simulink model "ClimaStopStrtReqMgr"  (R2022b)
settings: Solver=FixedStepDiscrete  StartTime=0.0  StopTime=10.0  FixedStep=1  RelTol=1e-3
settings Model: Creator=blue.ge  LastModifiedBy=blue.ge
settings DataIOCC: SaveFormat=StructureWithTime
settings OptimizationCC: DefaultParameterBehavior=Inlined  EnhancedBackFolding=on  PassReuseOutputArgsAs=Individual arguments  ZeroExternalMemoryAtStartup=off  ZeroInternalMemoryAtStartup=off  OptimizeBlockOrder=speed  DifferentSizesBufferReuse=on  OptimizationCustomize=off  EfficientTunableParamExpr=on
settings DebuggingCC: ParameterTunabilityLossMsg=error
settings ModelReferenceCC: ModelReferenceNumInstancesAllowed=Single
settings SFSimCC: MATLABDynamicMemAlloc=off
settings RTWCC: Description=AUTOSAR  SystemTargetFile=autosar.tlc  GenCodeOnly=on  TemplateMakefile=ert_default_tmf
settings CodeAppCC: ForceParamTrailComments=on  IgnoreCustomStorageClasses=off  OperatorAnnotations=on  SimulinkDataObjDesc=on  SFDataObjDesc=on  InsertBlockDesc=on
settings STFCustomTargetCC: TargetLangStandard=C99 (ISO)  MultiwordLength=256  ParMdlRefBuildCompliant=on  CompOptLevelCompliant=on  IncludeMdlTerminateFcn=off  CombineOutputUpdateFcns=on  SuppressErrorStatus=on  ERTCustomFileBanners=on  SupportNonFinite=off  SupportComplex=off  SupportContinuousTime=off  SupportNonInlinedSFcns=off  RemoveResetFunc=on  MATLABClassNameForMDSCustomization=Simulink.SoftwareTarget.ERTCustomization  AutosarCompliant=on  UseToolchainInfoCompliant=on  LookupTableObjectStructAxisOrder=2,1,3,4,...  LUTObjectStructOrderEvenSpacing=Size,Table,Breakpoints  SystemTargetFile=autosar.tlc
settings ERTTargetCC: TargetLangStandard=C99 (ISO)  ConcurrentExecutionCompliant=off  IncludeMdlTerminateFcn=off  SuppressErrorStatus=on  SupportNonFinite=off  SupportComplex=off  ModelStepFunctionPrototypeControlCompliant=off  CPPClassGenCompliant=off  AutosarCompliant=on  LookupTableObjectStructAxisOrder=2,1,3,4,...  LUTObjectStructOrderEvenSpacing=Size,Table,Breakpoints  InlinedParameterPlacement=NonHierarchical  ERTCustomFileTemplate=example_file_process.tlc

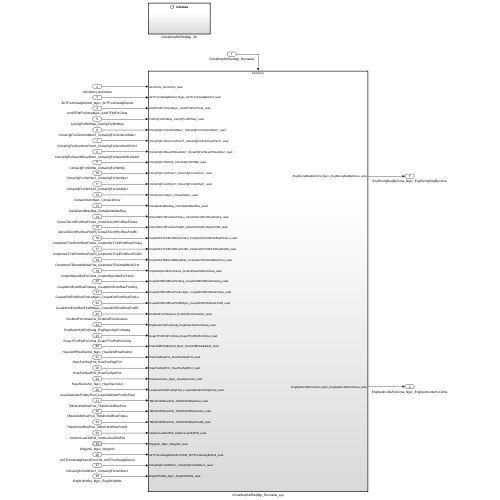
[thumbnail: Simulink's stored preview of the top-level diagram]
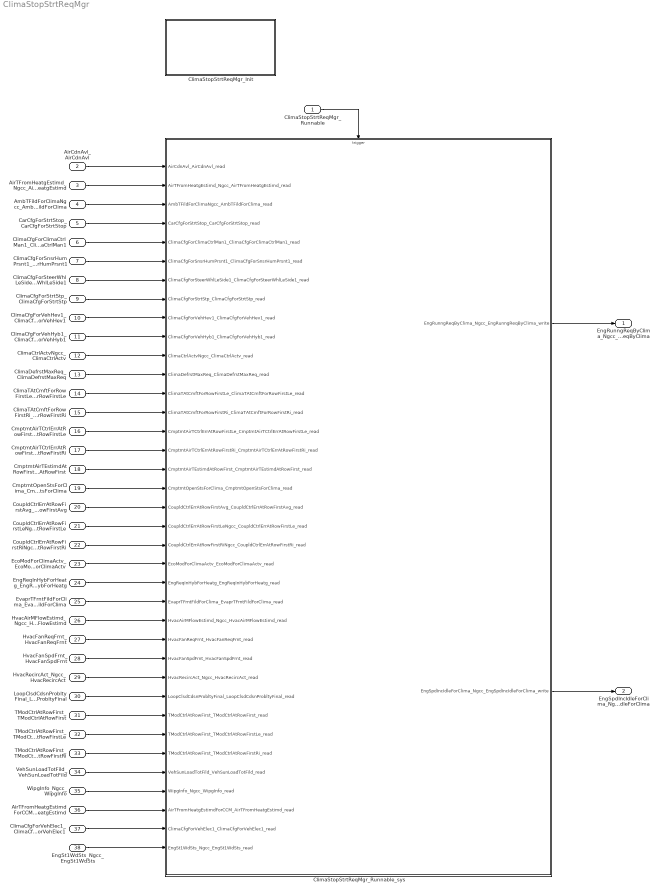
== system ClimaStopStrtReqMgr ==
  ClimaStopStrtReqMgr_Runnable : Inport  SampleTime=1  OutputFunctionCall=on
  AirCdnAvl_AirCdnAvl : Inport  Port=2  OutDataTypeStr=Boolean  PortDimensions=1
  AirTFromHeatgEstimd_Ngcc_AirTFromHeatgEstimd : Inport  Port=3  OutDataTypeStr=T30  PortDimensions=1
  AmbTFildForClimaNgcc_AmbTFildForClima : Inport  Port=4  OutDataTypeStr=T30  PortDimensions=1
  CarCfgForStrtStop_CarCfgForStrtStop : Inport  Port=5  OutDataTypeStr=Enum: CarCfgForStrtStop  PortDimensions=1
  ClimaCfgForClimaCtrlMan1_ClimaCfgForClimaCtrlMan1 : Inport  Port=6  OutDataTypeStr=Boolean  PortDimensions=1
  ClimaCfgForSnsrHumPrsnt1_ClimaCfgForSnsrHumPrsnt1 : Inport  Port=7  OutDataTypeStr=Boolean  PortDimensions=1
  ClimaCfgForSteerWhlLeSide1_ClimaCfgForSteerWhlLeSide1 : Inport  Port=8  OutDataTypeStr=Boolean  PortDimensions=1
  ClimaCfgForStrtStp_ClimaCfgForStrtStp : Inport  Port=9  OutDataTypeStr=Enum: CarCfgForStrtStop  PortDimensions=1
  ClimaCfgForVehHev1_ClimaCfgForVehHev1 : Inport  Port=10  OutDataTypeStr=Boolean  PortDimensions=1
  ClimaCfgForVehHyb1_ClimaCfgForVehHyb1 : Inport  Port=11  OutDataTypeStr=Boolean  PortDimensions=1
  ClimaCtrlActvNgcc_ClimaCtrlActv : Inport  Port=12  OutDataTypeStr=Boolean  PortDimensions=1
  ClimaDefrstMaxReq_ClimaDefrstMaxReq : Inport  Port=13  OutDataTypeStr=Boolean  PortDimensions=1
  ClimaTAtCmftForRowFirstLe_ClimaTAtCmftForRowFirstLe : Inport  Port=14  OutDataTypeStr=T30  PortDimensions=1
  ClimaTAtCmftForRowFirstRi_ClimaTAtCmftForRowFirstRi : Inport  Port=15  OutDataTypeStr=T30  PortDimensions=1
  CmptmtAirTCtrlErrAtRowFirstLe_CmptmtAirTCtrlErrAtRowFirstLe : Inport  Port=16  OutDataTypeStr=T30  PortDimensions=1
  CmptmtAirTCtrlErrAtRowFirstRi_CmptmtAirTCtrlErrAtRowFirstRi : Inport  Port=17  OutDataTypeStr=T30  PortDimensions=1
  CmptmtAirTEstimdAtRowFirst_CmptmtAirTEstimdAtRowFirst : Inport  Port=18  OutDataTypeStr=T30  PortDimensions=1
  CmptmtOpenStsForClima_CmptmtOpenStsForClima : Inport  Port=19  OutDataTypeStr=Boolean  PortDimensions=1
  CoupldCtrlErrAtRowFirstAvg_CoupldCtrlErrAtRowFirstAvg : Inport  Port=20  OutDataTypeStr=T30  PortDimensions=1
  CoupldCtrlErrAtRowFirstLeNgcc_CoupldCtrlErrAtRowFirstLe : Inport  Port=21  OutDataTypeStr=T30  PortDimensions=1
  CoupldCtrlErrAtRowFirstRiNgcc_CoupldCtrlErrAtRowFirstRi : Inport  Port=22  OutDataTypeStr=T30  PortDimensions=1
  EcoModForClimaActv_EcoModForClimaActv : Inport  Port=23  OutDataTypeStr=Enum: OnOff1  PortDimensions=1
  EngReqInHybForHeatg_EngReqInHybForHeatg : Inport  Port=24  OutDataTypeStr=Boolean  PortDimensions=1
  EvaprTFrntFildForClima_EvaprTFrntFildForClima : Inport  Port=25  OutDataTypeStr=T30  PortDimensions=1
  HvacAirMFlowEstimd_Ngcc_HvacAirMFlowEstimd : Inport  Port=26  OutMin=0  OutMax=1000  OutDataTypeStr=MFlow1  PortDimensions=1
  HvacFanReqFrnt_HvacFanReqFrnt : Inport  Port=27  OutDataTypeStr=HvacFanSpd  PortDimensions=1
  HvacFanSpdFrnt_HvacFanSpdFrnt : Inport  Port=28  OutDataTypeStr=HvacFanSpd  PortDimensions=1
  HvacRecircAct_Ngcc_HvacRecircAct : Inport  Port=29  OutDataTypeStr=Perc4  PortDimensions=1
  LoopClsdCdsnProbltyFinal_LoopClsdCdsnProbltyFinal : Inport  Port=30  OutDataTypeStr=Fac30  PortDimensions=1
  TModCtrlAtRowFirst_TModCtrlAtRowFirst : Inport  Port=31  OutDataTypeStr=T31  PortDimensions=1
  TModCtrlAtRowFirst_TModCtrlAtRowFirstLe : Inport  Port=32  OutDataTypeStr=T31  PortDimensions=1
  TModCtrlAtRowFirst_TModCtrlAtRowFirstRi : Inport  Port=33  OutDataTypeStr=T31  PortDimensions=1
  VehSunLoadTotFild_VehSunLoadTotFild : Inport  Port=34  OutDataTypeStr=SunLoad30  PortDimensions=1
  WipgInfo_Ngcc_WipgInfo : Inport  Port=35  OutDataTypeStr=Bus: WipgInfo  PortDimensions=1  BusOutputAsStruct=on
  AirTFromHeatgEstimdForCCM_AirTFromHeatgEstimd : Inport  Port=36  OutDataTypeStr=T30  PortDimensions=1
  ClimaCfgForVehElec1_ClimaCfgForVehElec1 : Inport  Port=37  OutDataTypeStr=Boolean  PortDimensions=1
  EngSt1WdSts_Ngcc_EngSt1WdSts : Inport  Port=38  OutDataTypeStr=Enum: EngSt1  PortDimensions=1
  ClimaStopStrtReqMgr_Init : Subsystem
  ClimaStopStrtReqMgr_Runnable_sys : Subsystem
  EngRunngReqByClima_Ngcc_EngRunngReqByClima : Outport  OutDataTypeStr=Enum: EngRunngReq  PortDimensions=1
  EngSpdIncIdleForClima_Ngcc_EngSpdIncIdleForClima : Outport  Port=2  OutDataTypeStr=Enum: Flg1  PortDimensions=1
  wires:
  AirCdnAvl_AirCdnAvl/1 -> ClimaStopStrtReqMgr_Runnable_sys/1(AirCdnAvl_AirCdnAvl_read)
  AirTFromHeatgEstimd_Ngcc_AirTFromHeatgEstimd/1 -> ClimaStopStrtReqMgr_Runnable_sys/2(AirTFromHeatgEstimd_Ngcc_AirTFromHeatgEstimd_read)
  AmbTFildForClimaNgcc_AmbTFildForClima/1 -> ClimaStopStrtReqMgr_Runnable_sys/3(AmbTFildForClimaNgcc_AmbTFildForClima_read)
  CarCfgForStrtStop_CarCfgForStrtStop/1 -> ClimaStopStrtReqMgr_Runnable_sys/4(CarCfgForStrtStop_CarCfgForStrtStop_read)
  ClimaCfgForClimaCtrlMan1_ClimaCfgForClimaCtrlMan1/1 -> ClimaStopStrtReqMgr_Runnable_sys/5(ClimaCfgForClimaCtrlMan1_ClimaCfgForClimaCtrlMan1_read)
  ClimaCfgForSnsrHumPrsnt1_ClimaCfgForSnsrHumPrsnt1/1 -> ClimaStopStrtReqMgr_Runnable_sys/6(ClimaCfgForSnsrHumPrsnt1_ClimaCfgForSnsrHumPrsnt1_read)
  ClimaCfgForSteerWhlLeSide1_ClimaCfgForSteerWhlLeSide1/1 -> ClimaStopStrtReqMgr_Runnable_sys/7(ClimaCfgForSteerWhlLeSide1_ClimaCfgForSteerWhlLeSide1_read)
  ClimaCfgForStrtStp_ClimaCfgForStrtStp/1 -> ClimaStopStrtReqMgr_Runnable_sys/8(ClimaCfgForStrtStp_ClimaCfgForStrtStp_read)
  ClimaCfgForVehHev1_ClimaCfgForVehHev1/1 -> ClimaStopStrtReqMgr_Runnable_sys/9(ClimaCfgForVehHev1_ClimaCfgForVehHev1_read)
  ClimaCfgForVehHyb1_ClimaCfgForVehHyb1/1 -> ClimaStopStrtReqMgr_Runnable_sys/10(ClimaCfgForVehHyb1_ClimaCfgForVehHyb1_read)
  ClimaCtrlActvNgcc_ClimaCtrlActv/1 -> ClimaStopStrtReqMgr_Runnable_sys/11(ClimaCtrlActvNgcc_ClimaCtrlActv_read)
  ClimaDefrstMaxReq_ClimaDefrstMaxReq/1 -> ClimaStopStrtReqMgr_Runnable_sys/12(ClimaDefrstMaxReq_ClimaDefrstMaxReq_read)
  ClimaTAtCmftForRowFirstLe_ClimaTAtCmftForRowFirstLe/1 -> ClimaStopStrtReqMgr_Runnable_sys/13(ClimaTAtCmftForRowFirstLe_ClimaTAtCmftForRowFirstLe_read)
  ClimaTAtCmftForRowFirstRi_ClimaTAtCmftForRowFirstRi/1 -> ClimaStopStrtReqMgr_Runnable_sys/14(ClimaTAtCmftForRowFirstRi_ClimaTAtCmftForRowFirstRi_read)
  CmptmtAirTCtrlErrAtRowFirstLe_CmptmtAirTCtrlErrAtRowFirstLe/1 -> ClimaStopStrtReqMgr_Runnable_sys/15(CmptmtAirTCtrlErrAtRowFirstLe_CmptmtAirTCtrlErrAtRowFirstLe_read)
  CmptmtAirTCtrlErrAtRowFirstRi_CmptmtAirTCtrlErrAtRowFirstRi/1 -> ClimaStopStrtReqMgr_Runnable_sys/16(CmptmtAirTCtrlErrAtRowFirstRi_CmptmtAirTCtrlErrAtRowFirstRi_read)
  CmptmtAirTEstimdAtRowFirst_CmptmtAirTEstimdAtRowFirst/1 -> ClimaStopStrtReqMgr_Runnable_sys/17(CmptmtAirTEstimdAtRowFirst_CmptmtAirTEstimdAtRowFirst_read)
  CmptmtOpenStsForClima_CmptmtOpenStsForClima/1 -> ClimaStopStrtReqMgr_Runnable_sys/18(CmptmtOpenStsForClima_CmptmtOpenStsForClima_read)
  CoupldCtrlErrAtRowFirstAvg_CoupldCtrlErrAtRowFirstAvg/1 -> ClimaStopStrtReqMgr_Runnable_sys/19(CoupldCtrlErrAtRowFirstAvg_CoupldCtrlErrAtRowFirstAvg_read)
  CoupldCtrlErrAtRowFirstLeNgcc_CoupldCtrlErrAtRowFirstLe/1 -> ClimaStopStrtReqMgr_Runnable_sys/20(CoupldCtrlErrAtRowFirstLeNgcc_CoupldCtrlErrAtRowFirstLe_read)
  CoupldCtrlErrAtRowFirstRiNgcc_CoupldCtrlErrAtRowFirstRi/1 -> ClimaStopStrtReqMgr_Runnable_sys/21(CoupldCtrlErrAtRowFirstRiNgcc_CoupldCtrlErrAtRowFirstRi_read)
  EcoModForClimaActv_EcoModForClimaActv/1 -> ClimaStopStrtReqMgr_Runnable_sys/22(EcoModForClimaActv_EcoModForClimaActv_read)
  EngReqInHybForHeatg_EngReqInHybForHeatg/1 -> ClimaStopStrtReqMgr_Runnable_sys/23(EngReqInHybForHeatg_EngReqInHybForHeatg_read)
  EvaprTFrntFildForClima_EvaprTFrntFildForClima/1 -> ClimaStopStrtReqMgr_Runnable_sys/24(EvaprTFrntFildForClima_EvaprTFrntFildForClima_read)
  HvacAirMFlowEstimd_Ngcc_HvacAirMFlowEstimd/1 -> ClimaStopStrtReqMgr_Runnable_sys/25(HvacAirMFlowEstimd_Ngcc_HvacAirMFlowEstimd_read)
  HvacFanReqFrnt_HvacFanReqFrnt/1 -> ClimaStopStrtReqMgr_Runnable_sys/26(HvacFanReqFrnt_HvacFanReqFrnt_read)
  HvacFanSpdFrnt_HvacFanSpdFrnt/1 -> ClimaStopStrtReqMgr_Runnable_sys/27(HvacFanSpdFrnt_HvacFanSpdFrnt_read)
  HvacRecircAct_Ngcc_HvacRecircAct/1 -> ClimaStopStrtReqMgr_Runnable_sys/28(HvacRecircAct_Ngcc_HvacRecircAct_read)
  LoopClsdCdsnProbltyFinal_LoopClsdCdsnProbltyFinal/1 -> ClimaStopStrtReqMgr_Runnable_sys/29(LoopClsdCdsnProbltyFinal_LoopClsdCdsnProbltyFinal_read)
  TModCtrlAtRowFirst_TModCtrlAtRowFirst/1 -> ClimaStopStrtReqMgr_Runnable_sys/30(TModCtrlAtRowFirst_TModCtrlAtRowFirst_read)
  TModCtrlAtRowFirst_TModCtrlAtRowFirstLe/1 -> ClimaStopStrtReqMgr_Runnable_sys/31(TModCtrlAtRowFirst_TModCtrlAtRowFirstLe_read)
  TModCtrlAtRowFirst_TModCtrlAtRowFirstRi/1 -> ClimaStopStrtReqMgr_Runnable_sys/32(TModCtrlAtRowFirst_TModCtrlAtRowFirstRi_read)
  VehSunLoadTotFild_VehSunLoadTotFild/1 -> ClimaStopStrtReqMgr_Runnable_sys/33(VehSunLoadTotFild_VehSunLoadTotFild_read)
  WipgInfo_Ngcc_WipgInfo/1 -> ClimaStopStrtReqMgr_Runnable_sys/34(WipgInfo_Ngcc_WipgInfo_read)
  AirTFromHeatgEstimdForCCM_AirTFromHeatgEstimd/1 -> ClimaStopStrtReqMgr_Runnable_sys/35(AirTFromHeatgEstimdForCCM_AirTFromHeatgEstimd_read)
  ClimaCfgForVehElec1_ClimaCfgForVehElec1/1 -> ClimaStopStrtReqMgr_Runnable_sys/36(ClimaCfgForVehElec1_ClimaCfgForVehElec1_read)
  EngSt1WdSts_Ngcc_EngSt1WdSts/1 -> ClimaStopStrtReqMgr_Runnable_sys/37(EngSt1WdSts_Ngcc_EngSt1WdSts_read)
  ClimaStopStrtReqMgr_Runnable_sys/1(EngRunngReqByClima_Ngcc_EngRunngReqByClima_write) -> EngRunngReqByClima_Ngcc_EngRunngReqByClima/1
  ClimaStopStrtReqMgr_Runnable_sys/2(EngSpdIncIdleForClima_Ngcc_EngSpdIncIdleForClima_write) -> EngSpdIncIdleForClima_Ngcc_EngSpdIncIdleForClima/1
  ClimaStopStrtReqMgr_Runnable/1 -> ClimaStopStrtReqMgr_Runnable_sys/Trigger

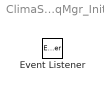
== system ClimaStopStrtReqMgr/ClimaStopStrtReqMgr_Init ==
  Event Listener : EventListener  EventName=reset

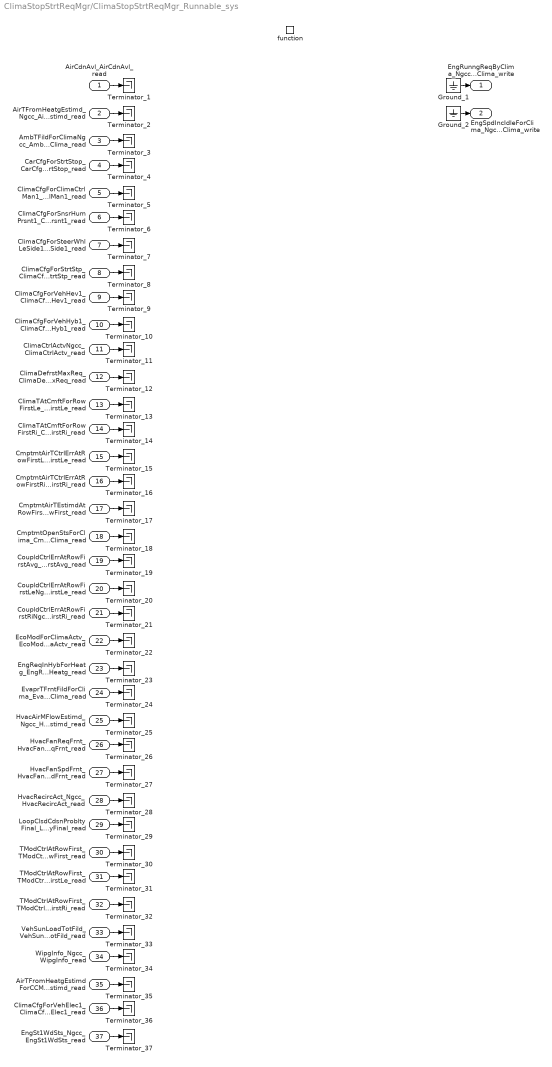
== system ClimaStopStrtReqMgr/ClimaStopStrtReqMgr_Runnable_sys ==
  AirCdnAvl_AirCdnAvl_read : Inport
  AirTFromHeatgEstimd_Ngcc_AirTFromHeatgEstimd_read : Inport  Port=2
  AmbTFildForClimaNgcc_AmbTFildForClima_read : Inport  Port=3
  CarCfgForStrtStop_CarCfgForStrtStop_read : Inport  Port=4
  ClimaCfgForClimaCtrlMan1_ClimaCfgForClimaCtrlMan1_read : Inport  Port=5
  ClimaCfgForSnsrHumPrsnt1_ClimaCfgForSnsrHumPrsnt1_read : Inport  Port=6
  ClimaCfgForSteerWhlLeSide1_ClimaCfgForSteerWhlLeSide1_read : Inport  Port=7
  ClimaCfgForStrtStp_ClimaCfgForStrtStp_read : Inport  Port=8
  ClimaCfgForVehHev1_ClimaCfgForVehHev1_read : Inport  Port=9
  ClimaCfgForVehHyb1_ClimaCfgForVehHyb1_read : Inport  Port=10
  ClimaCtrlActvNgcc_ClimaCtrlActv_read : Inport  Port=11
  ClimaDefrstMaxReq_ClimaDefrstMaxReq_read : Inport  Port=12
  ClimaTAtCmftForRowFirstLe_ClimaTAtCmftForRowFirstLe_read : Inport  Port=13
  ClimaTAtCmftForRowFirstRi_ClimaTAtCmftForRowFirstRi_read : Inport  Port=14
  CmptmtAirTCtrlErrAtRowFirstLe_CmptmtAirTCtrlErrAtRowFirstLe_read : Inport  Port=15
  CmptmtAirTCtrlErrAtRowFirstRi_CmptmtAirTCtrlErrAtRowFirstRi_read : Inport  Port=16
  CmptmtAirTEstimdAtRowFirst_CmptmtAirTEstimdAtRowFirst_read : Inport  Port=17
  CmptmtOpenStsForClima_CmptmtOpenStsForClima_read : Inport  Port=18
  CoupldCtrlErrAtRowFirstAvg_CoupldCtrlErrAtRowFirstAvg_read : Inport  Port=19
  CoupldCtrlErrAtRowFirstLeNgcc_CoupldCtrlErrAtRowFirstLe_read : Inport  Port=20
  CoupldCtrlErrAtRowFirstRiNgcc_CoupldCtrlErrAtRowFirstRi_read : Inport  Port=21
  EcoModForClimaActv_EcoModForClimaActv_read : Inport  Port=22
  EngReqInHybForHeatg_EngReqInHybForHeatg_read : Inport  Port=23
  EvaprTFrntFildForClima_EvaprTFrntFildForClima_read : Inport  Port=24
  HvacAirMFlowEstimd_Ngcc_HvacAirMFlowEstimd_read : Inport  Port=25
  HvacFanReqFrnt_HvacFanReqFrnt_read : Inport  Port=26
  HvacFanSpdFrnt_HvacFanSpdFrnt_read : Inport  Port=27
  HvacRecircAct_Ngcc_HvacRecircAct_read : Inport  Port=28
  LoopClsdCdsnProbltyFinal_LoopClsdCdsnProbltyFinal_read : Inport  Port=29
  TModCtrlAtRowFirst_TModCtrlAtRowFirst_read : Inport  Port=30
  TModCtrlAtRowFirst_TModCtrlAtRowFirstLe_read : Inport  Port=31
  TModCtrlAtRowFirst_TModCtrlAtRowFirstRi_read : Inport  Port=32
  VehSunLoadTotFild_VehSunLoadTotFild_read : Inport  Port=33
  WipgInfo_Ngcc_WipgInfo_read : Inport  Port=34
  AirTFromHeatgEstimdForCCM_AirTFromHeatgEstimd_read : Inport  Port=35
  ClimaCfgForVehElec1_ClimaCfgForVehElec1_read : Inport  Port=36
  EngSt1WdSts_Ngcc_EngSt1WdSts_read : Inport  Port=37
  function : TriggerPort  TriggerType=function-call  VariantControl=(inherit)  SampleTimeType=periodic  SampleTime=-1
  Ground_1 : Ground
  Ground_2 : Ground
  Terminator_{1..37} (37 alike) : Terminator
  EngRunngReqByClima_Ngcc_EngRunngReqByClima_write : Outport
      settings: EnsureOutportIsVirtual=on
  EngSpdIncIdleForClima_Ngcc_EngSpdIncIdleForClima_write : Outport  Port=2
      settings: EnsureOutportIsVirtual=on
  wires:
  AirCdnAvl_AirCdnAvl_read/1 -> Terminator_1/1
  AirTFromHeatgEstimd_Ngcc_AirTFromHeatgEstimd_read/1 -> Terminator_2/1
  AmbTFildForClimaNgcc_AmbTFildForClima_read/1 -> Terminator_3/1
  CarCfgForStrtStop_CarCfgForStrtStop_read/1 -> Terminator_4/1
  ClimaCfgForClimaCtrlMan1_ClimaCfgForClimaCtrlMan1_read/1 -> Terminator_5/1
  ClimaCfgForSnsrHumPrsnt1_ClimaCfgForSnsrHumPrsnt1_read/1 -> Terminator_6/1
  ClimaCfgForSteerWhlLeSide1_ClimaCfgForSteerWhlLeSide1_read/1 -> Terminator_7/1
  ClimaCfgForStrtStp_ClimaCfgForStrtStp_read/1 -> Terminator_8/1
  ClimaCfgForVehHev1_ClimaCfgForVehHev1_read/1 -> Terminator_9/1
  ClimaCfgForVehHyb1_ClimaCfgForVehHyb1_read/1 -> Terminator_10/1
  ClimaCtrlActvNgcc_ClimaCtrlActv_read/1 -> Terminator_11/1
  ClimaDefrstMaxReq_ClimaDefrstMaxReq_read/1 -> Terminator_12/1
  ClimaTAtCmftForRowFirstLe_ClimaTAtCmftForRowFirstLe_read/1 -> Terminator_13/1
  ClimaTAtCmftForRowFirstRi_ClimaTAtCmftForRowFirstRi_read/1 -> Terminator_14/1
  CmptmtAirTCtrlErrAtRowFirstLe_CmptmtAirTCtrlErrAtRowFirstLe_read/1 -> Terminator_15/1
  CmptmtAirTCtrlErrAtRowFirstRi_CmptmtAirTCtrlErrAtRowFirstRi_read/1 -> Terminator_16/1
  CmptmtAirTEstimdAtRowFirst_CmptmtAirTEstimdAtRowFirst_read/1 -> Terminator_17/1
  CmptmtOpenStsForClima_CmptmtOpenStsForClima_read/1 -> Terminator_18/1
  CoupldCtrlErrAtRowFirstAvg_CoupldCtrlErrAtRowFirstAvg_read/1 -> Terminator_19/1
  CoupldCtrlErrAtRowFirstLeNgcc_CoupldCtrlErrAtRowFirstLe_read/1 -> Terminator_20/1
  CoupldCtrlErrAtRowFirstRiNgcc_CoupldCtrlErrAtRowFirstRi_read/1 -> Terminator_21/1
  EcoModForClimaActv_EcoModForClimaActv_read/1 -> Terminator_22/1
  EngReqInHybForHeatg_EngReqInHybForHeatg_read/1 -> Terminator_23/1
  EvaprTFrntFildForClima_EvaprTFrntFildForClima_read/1 -> Terminator_24/1
  HvacAirMFlowEstimd_Ngcc_HvacAirMFlowEstimd_read/1 -> Terminator_25/1
  HvacFanReqFrnt_HvacFanReqFrnt_read/1 -> Terminator_26/1
  HvacFanSpdFrnt_HvacFanSpdFrnt_read/1 -> Terminator_27/1
  HvacRecircAct_Ngcc_HvacRecircAct_read/1 -> Terminator_28/1
  LoopClsdCdsnProbltyFinal_LoopClsdCdsnProbltyFinal_read/1 -> Terminator_29/1
  TModCtrlAtRowFirst_TModCtrlAtRowFirst_read/1 -> Terminator_30/1
  TModCtrlAtRowFirst_TModCtrlAtRowFirstLe_read/1 -> Terminator_31/1
  TModCtrlAtRowFirst_TModCtrlAtRowFirstRi_read/1 -> Terminator_32/1
  VehSunLoadTotFild_VehSunLoadTotFild_read/1 -> Terminator_33/1
  WipgInfo_Ngcc_WipgInfo_read/1 -> Terminator_34/1
  AirTFromHeatgEstimdForCCM_AirTFromHeatgEstimd_read/1 -> Terminator_35/1
  ClimaCfgForVehElec1_ClimaCfgForVehElec1_read/1 -> Terminator_36/1
  EngSt1WdSts_Ngcc_EngSt1WdSts_read/1 -> Terminator_37/1
  Ground_1/1 -> EngRunngReqByClima_Ngcc_EngRunngReqByClima_write/1
  Ground_2/1 -> EngSpdIncIdleForClima_Ngcc_EngSpdIncIdleForClima_write/1
